simulink model "CCMLin2Mgr"  (R2022b)
settings: Solver=FixedStepDiscrete  StartTime=0.0  StopTime=10.0  FixedStep=0.02  RelTol=1e-3
undefined symbols (not defined in the file or in its folder): ComChlType, NetworkChlType, P_LinIfSchHandleType, P_Std_ReturnType
(part 1 of 3)
settings Model: Creator=blue.ge  LastModifiedBy=blue.ge
settings DataIOCC: SaveFormat=StructureWithTime
settings OptimizationCC: DefaultParameterBehavior=Inlined  EnhancedBackFolding=on  PassReuseOutputArgsAs=Individual arguments  ZeroExternalMemoryAtStartup=off  ZeroInternalMemoryAtStartup=off  OptimizeBlockOrder=speed  DifferentSizesBufferReuse=on  OptimizationCustomize=off  EfficientTunableParamExpr=on
settings DebuggingCC: ParameterTunabilityLossMsg=error
settings ModelReferenceCC: ModelReferenceNumInstancesAllowed=Single
settings SFSimCC: MATLABDynamicMemAlloc=off
settings RTWCC: Description=AUTOSAR  SystemTargetFile=autosar.tlc  GenCodeOnly=on  TemplateMakefile=ert_default_tmf
settings CodeAppCC: ForceParamTrailComments=on  IgnoreCustomStorageClasses=off  OperatorAnnotations=on  SimulinkDataObjDesc=on  SFDataObjDesc=on  InsertBlockDesc=on
settings STFCustomTargetCC: TargetLangStandard=C99 (ISO)  MultiwordLength=256  ParMdlRefBuildCompliant=on  CompOptLevelCompliant=on  IncludeMdlTerminateFcn=off  CombineOutputUpdateFcns=on  SuppressErrorStatus=on  ERTCustomFileBanners=on  SupportNonFinite=off  SupportComplex=off  SupportContinuousTime=off  SupportNonInlinedSFcns=off  RemoveResetFunc=on  MATLABClassNameForMDSCustomization=Simulink.SoftwareTarget.ERTCustomization  AutosarCompliant=on  UseToolchainInfoCompliant=on  LookupTableObjectStructAxisOrder=2,1,3,4,...  LUTObjectStructOrderEvenSpacing=Size,Table,Breakpoints  SystemTargetFile=autosar.tlc
settings ERTTargetCC: TargetLangStandard=C99 (ISO)  ConcurrentExecutionCompliant=off  IncludeMdlTerminateFcn=off  SuppressErrorStatus=on  SupportNonFinite=off  SupportComplex=off  ModelStepFunctionPrototypeControlCompliant=off  CPPClassGenCompliant=off  AutosarCompliant=on  LookupTableObjectStructAxisOrder=2,1,3,4,...  LUTObjectStructOrderEvenSpacing=Size,Table,Breakpoints  InlinedParameterPlacement=NonHierarchical  ERTCustomFileTemplate=example_file_process.tlc

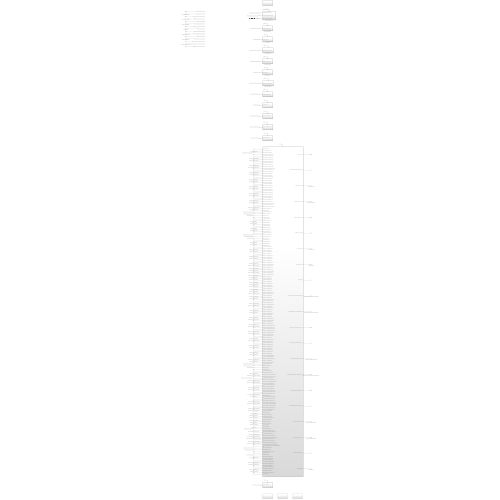
[thumbnail: Simulink's stored preview of the top-level diagram]
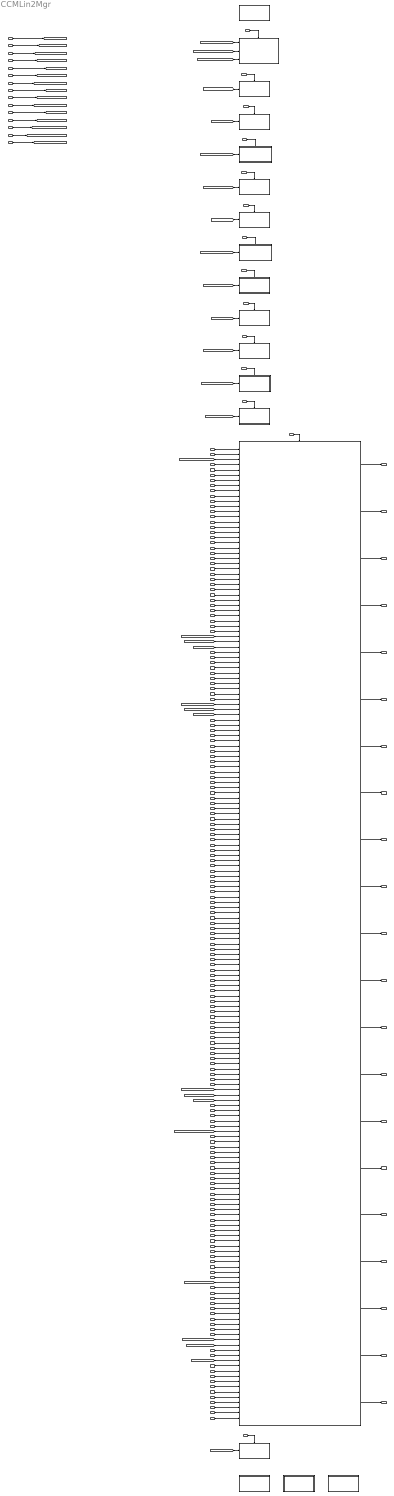
== system CCMLin2Mgr ==
  Lin2Mgr_AFUPartNo10Cmpl : Inport  OutputFunctionCall=on
  Lin2Mgr_AFUPartNoCmpl : Inport  Port=2  OutputFunctionCall=on
  Lin2Mgr_AFUSerNo : Inport  Port=3  OutputFunctionCall=on
  Lin2Mgr_AIUPartNo10Cmpl : Inport  Port=4  OutputFunctionCall=on
  Lin2Mgr_AIUPartNoCmpl : Inport  Port=5  OutputFunctionCall=on
  Lin2Mgr_AIUSerNo : Inport  Port=6  OutputFunctionCall=on
  Lin2Mgr_CDSPartNo10Cmpl : Inport  Port=7  OutputFunctionCall=on
  Lin2Mgr_CDSPartNoCmpl : Inport  Port=8  OutputFunctionCall=on
  Lin2Mgr_CDSSerNo : Inport  Port=9  OutputFunctionCall=on
  Lin2Mgr_KeepComActv : Inport  Port=10  SampleTime=0.02  OutputFunctionCall=on
  Lin2Mgr_PMSIPartNo10Hw : Inport  Port=11  OutputFunctionCall=on
  Lin2Mgr_PMSIPartNoHw : Inport  Port=12  OutputFunctionCall=on
  Lin2Mgr_Runnable : Inport  Port=13  SampleTime=0.1  OutputFunctionCall=on
  Lin2Mgr_SerNoPMSI : Inport  Port=14  OutputFunctionCall=on
  AFUPartNo10Cmpl_AFUPartNo10Cmpl : Inport  Port=15  OutDataTypeStr=Bus: PartNrNod10  PortDimensions=1  BusOutputAsStruct=on
  HvacCfgForClimaCtrl_HvacCfgForAQSTypes : Inport  Port=16  OutDataTypeStr=UInt8  PortDimensions=1
  SysServCarCfgSts_SysServCCPAQSSts : Inport  Port=17  OutDataTypeStr=UInt8  PortDimensions=1
  AFUPartNoCmpl_AFUPartNoCmpl : Inport  Port=18  OutDataTypeStr=Bus: PartNrNodLIN  PortDimensions=1  BusOutputAsStruct=on
  AFUSerNo_AFUSerNo : Inport  Port=19  OutDataTypeStr=Bus: NrSerlNodLIN  PortDimensions=1  BusOutputAsStruct=on
  AIUPartNo10Cmpl_AIUPartNo10Cmpl : Inport  Port=20  OutDataTypeStr=Bus: PartNrNod10  PortDimensions=1  BusOutputAsStruct=on
  AIUPartNoCmpl_AIUPartNoCmpl : Inport  Port=21  OutDataTypeStr=Bus: PartNrNodLIN  PortDimensions=1  BusOutputAsStruct=on
  AIUSerNo_AIUSerNo : Inport  Port=22  OutDataTypeStr=Bus: NrSerlNodLIN  PortDimensions=1  BusOutputAsStruct=on
  CDSPartNo10Cmpl_CDSPartNo10Cmpl : Inport  Port=23  OutDataTypeStr=Bus: PartNrNod10  PortDimensions=1  BusOutputAsStruct=on
  CDSPartNoCmpl_CDSPartNoCmpl : Inport  Port=24  OutDataTypeStr=Bus: PartNrNodLIN  PortDimensions=1  BusOutputAsStruct=on
  CDSSerNo_CDSSerNo : Inport  Port=25  OutDataTypeStr=Bus: NrSerlNodLIN  PortDimensions=1  BusOutputAsStruct=on
  KeepComActvL2_KeepComActvL2 : Inport  Port=26  OutDataTypeStr=Enum: OnOff1  PortDimensions=1
  PMSIPartNo10Hw_PMSIPartNo10Hw : Inport  Port=27  OutDataTypeStr=Bus: PartNrNod10  PortDimensions=1  BusOutputAsStruct=on
  PMSIPartNoHw_PMSIPartNoHw : Inport  Port=28  OutDataTypeStr=Bus: PartNrNodLIN  PortDimensions=1  BusOutputAsStruct=on
  RlyCmdCmft_RlyCmdCmft : Inport  Port=29  OutDataTypeStr=Enum: OnOff1  PortDimensions=1
  SysServCarCfgSts_SysServCarID : Inport  Port=30  OutDataTypeStr=Enum: SysServCarID  PortDimensions=1
  SysServCarCfgSts_SysServCCPHEXSensorSts : Inport  Port=31  OutDataTypeStr=UInt8  PortDimensions=1
  SysServCarCfgSts_SysServCCPLin2AFUSts : Inport  Port=32  OutDataTypeStr=Boolean  PortDimensions=1
  SysServCarCfgSts_SysServCCPLin2AIUSts : Inport  Port=33  OutDataTypeStr=UInt8  PortDimensions=1
  SysServCarCfgSts_SysServCCPLin2CDSSts : Inport  Port=34  OutDataTypeStr=Boolean  PortDimensions=1
  SysServCarCfgSts_SysServCCPLin2PMSISts : Inport  Port=35  OutDataTypeStr=Boolean  PortDimensions=1
  SysServCarCfgSts_SysServCCPLin3VGASts : Inport  Port=36  OutDataTypeStr=Boolean  PortDimensions=1
  SysServCarCfgSts_SysServDUAirVentSnrSts : Inport  Port=37  OutDataTypeStr=Boolean  PortDimensions=1
  SysServCarCfgSts_SysServDUBlowerPwmSignSts : Inport  Port=38  OutDataTypeStr=Boolean  PortDimensions=1
  SysServCarCfgSts_SysServDUBlowerPwmSignTypeSts : Inport  Port=39  OutDataTypeStr=UInt8  PortDimensions=1
  SysServCarCfgSts_SysServDUBlowerPwmSts : Inport  Port=40  OutDataTypeStr=Boolean  PortDimensions=1
  SysServCarCfgSts_SysServDUDCDefrostSts : Inport  Port=41  OutDataTypeStr=UInt8  PortDimensions=1
  SysServCarCfgSts_SysServDUDCModeSts : Inport  Port=42  OutDataTypeStr=UInt8  PortDimensions=1
  SysServCarCfgSts_SysServDUDCRecSts : Inport  Port=43  OutDataTypeStr=UInt8  PortDimensions=1
  SysServCarCfgSts_SysServDUDCSModeSts : Inport  Port=44  OutDataTypeStr=UInt8  PortDimensions=1
  SysServCarCfgSts_SysServDUDCSTLSts : Inport  Port=45  OutDataTypeStr=UInt8  PortDimensions=1
  SysServCarCfgSts_SysServDUDCTLSts : Inport  Port=46  OutDataTypeStr=UInt8  PortDimensions=1
  SysServCarCfgSts_SysServDUDCTRSts : Inport  Port=47  OutDataTypeStr=UInt8  PortDimensions=1
  SysServCarCfgSts_SysServDUFLFootSts : Inport  Port=48  OutDataTypeStr=UInt8  PortDimensions=1
  SysServCarCfgSts_SysServDUFLVentSts : Inport  Port=49  OutDataTypeStr=UInt8  PortDimensions=1
  SysServCarCfgSts_SysServDUFRFootSts : Inport  Port=50  OutDataTypeStr=UInt8  PortDimensions=1
  SysServCarCfgSts_SysServDUFRVentSts : Inport  Port=51  OutDataTypeStr=UInt8  PortDimensions=1
  SysServCarCfgSts_SysServDUIncarMotorSts : Inport  Port=52  OutDataTypeStr=Boolean  PortDimensions=1
  SysServCarCfgSts_SysServDULin1HBMFSts : Inport  Port=53  OutDataTypeStr=Boolean  PortDimensions=1
  SysServCarCfgSts_SysServDULin4HFAFSts : Inport  Port=54  OutDataTypeStr=UInt32  PortDimensions=1
  SysServCarCfgSts_SysServDUSFLFootSts : Inport  Port=55  OutDataTypeStr=UInt8  PortDimensions=1
  SysServCarCfgSts_SysServDUSFLVentSts : Inport  Port=56  OutDataTypeStr=UInt8  PortDimensions=1
  SysServCarCfgSts_SysServDUSFRFootSts : Inport  Port=57  OutDataTypeStr=UInt8  PortDimensions=1
  SysServCarCfgSts_SysServDUSFRVentSts : Inport  Port=58  OutDataTypeStr=UInt8  PortDimensions=1
  SysServCarCfgSts_SysServDUSnsrForFCmptmtTSts : Inport  Port=59  OutDataTypeStr=UInt8  PortDimensions=1
  SysServCarCfgSts_SysServDUSnsrForRair : Inport  Port=60  OutDataTypeStr=UInt8  PortDimensions=1
  SysServCarCfgSts_SysServDUSnsrForRCmptmtTSts : Inport  Port=61  OutDataTypeStr=UInt8  PortDimensions=1
  AACAirClngErrSts_AACAirClngErrSts : Inport  Port=62  OutDataTypeStr=Enum: AACAirClngErrSts  PortDimensions=1
  AFUGenErr_AFUGenErr : Inport  Port=63  OutDataTypeStr=Enum: OnOff1  PortDimensions=1
  AirClngErrSts_AirClngErrSts : Inport  Port=64  OutDataTypeStr=Enum: AirClngErrSts  PortDimensions=1
  AirFragFanErr_AirFragFanErr : Inport  Port=65  OutDataTypeStr=Enum: Err1  PortDimensions=1
  AirFragMot1Err_AirFragMot1Err : Inport  Port=66  OutDataTypeStr=Enum: Err1  PortDimensions=1
  AirFragMot2Err_AirFragMot2Err : Inport  Port=67  OutDataTypeStr=Enum: Err1  PortDimensions=1
  AirFragMot3Err_AirFragMot3Err : Inport  Port=68  OutDataTypeStr=Enum: Err1  PortDimensions=1
  AirFragMot4Err_AirFragMot4Err : Inport  Port=69  OutDataTypeStr=Enum: Err1  PortDimensions=1
  AirFragMot5Err_AirFragMot5Err : Inport  Port=70  OutDataTypeStr=Enum: Err1  PortDimensions=1
  AirFragOvrVoltErr_AirFragOvrVoltErr : Inport  Port=71  OutDataTypeStr=Enum: Err1  PortDimensions=1
  AirFragTempErr_AirFragTempErr : Inport  Port=72  OutDataTypeStr=Enum: TempErr  PortDimensions=1
  AirFragUndVoltErr_AirFragUndVoltErr : Inport  Port=73  OutDataTypeStr=Enum: Err1  PortDimensions=1
  AQSAirQuality_AQSAirQuality : Inport  Port=74  OutDataTypeStr=Enum: AQSAirQuality  PortDimensions=1
  AQSCOxstatus_AQSCOxstatus : Inport  Port=75  OutDataTypeStr=Enum: AQSAirQuality  PortDimensions=1
  AQSNH3status_AQSNH3status : Inport  Port=76  OutDataTypeStr=Enum: AQSAirQuality  PortDimensions=1
  AQSNoxStatus_AQSNoxStatus : Inport  Port=77  OutDataTypeStr=Enum: AQSAirQuality  PortDimensions=1
  CarCfgForClimaCtrl_CarCfgForAirClng : Inport  Port=78  OutDataTypeStr=Enum: CarCfgForAirClng  PortDimensions=1
  CarCfgForClimaCtrl_CarCfgForAirClnrAdv : Inport  Port=79  OutDataTypeStr=UInt8  PortDimensions=1
  CarCfgForClimaCtrl_CarCfgForAirFrag : Inport  Port=80  OutDataTypeStr=Enum: CarCfgForAirFrag  PortDimensions=1
  CarCfgForClimaCtrl_CarCfgForAirMtrlSnsr : Inport  Port=81  OutDataTypeStr=Enum: CarCfgForAirMtrlSnsrGly  PortDimensions=1
  CarCfgForClimaCtrl_CarCfgForAirQly : Inport  Port=82  OutDataTypeStr=Enum: CarCfgForAirQlyGly  PortDimensions=1
  CarCfgForClimaCtrl_CarCfgForAmntOfSeat : Inport  Port=83  OutDataTypeStr=Enum: CarCfgForAmntOfSeatTyp3  PortDimensions=1
  CarCfgForClimaCtrl_CarCfgForAutDemst : Inport  Port=84  OutDataTypeStr=UInt8  PortDimensions=1
  CarCfgForClimaCtrl_CarCfgForAuxWtrPmp : Inport  Port=85  OutDataTypeStr=Enum: CarCfgForAuxWtrPmp  PortDimensions=1
  CarCfgForClimaCtrl_CarCfgForBRAND : Inport  Port=86  OutDataTypeStr=UInt8  PortDimensions=1
  CarCfgForClimaCtrl_CarCfgForBypVlvInHvac : Inport  Port=87  OutDataTypeStr=Enum: CarCfgForBypVlvInHvac2  PortDimensions=1
  CarCfgForClimaCtrl_CarCfgForCabinOxgenSupply : Inport  Port=88  OutDataTypeStr=UInt8  PortDimensions=1
  CarCfgForClimaCtrl_CarCfgForClsVlvInHvac : Inport  Port=89  OutDataTypeStr=Enum: CarCfgForClsVlvInHvac  PortDimensions=1
  CarCfgForClimaCtrl_CarCfgForCmprAcTyp : Inport  Port=90  OutDataTypeStr=UInt8  PortDimensions=1
  CarCfgForClimaCtrl_CarCfgForCmptmtClng : Inport  Port=91  OutDataTypeStr=Enum: CarCfgForCmptmtClng  PortDimensions=1
  CarCfgForClimaCtrl_CarCfgForElectricLiftingModule : Inport  Port=92  OutDataTypeStr=UInt8  PortDimensions=1
  CarCfgForClimaCtrl_CarCfgForElecVentAdjmt : Inport  Port=93  OutDataTypeStr=Enum: CarCfgForElecVentAdjmt  PortDimensions=1
  CarCfgForClimaCtrl_CarCfgForExtVentnClimaCtrl : Inport  Port=94  OutDataTypeStr=Enum: CarCfgForExtVentnClimaCtrl  PortDimensions=1
  CarCfgForClimaCtrl_CarCfgForFlcTyp : Inport  Port=95  OutDataTypeStr=Enum: CarCfgForFlcTyp  PortDimensions=1
  CarCfgForClimaCtrl_CarCfgForFoldCSD : Inport  Port=96  OutDataTypeStr=Enum: CarCfgForFoldCSD  PortDimensions=1
  CarCfgForClimaCtrl_CarCfgForFuTyp : Inport  Port=97  OutDataTypeStr=Enum: CarCfgForFuTyp  PortDimensions=1
  CarCfgForClimaCtrl_CarCfgForGlassTyp : Inport  Port=98  OutDataTypeStr=Enum: CarCfgForGlassTyp  PortDimensions=1
  CarCfgForClimaCtrl_CarCfgForHeadRadar : Inport  Port=99  OutDataTypeStr=Enum: HeadRadar  PortDimensions=1
  CarCfgForClimaCtrl_CarCfgForHeatPmp : Inport  Port=100  OutDataTypeStr=Enum: CarCfgForHeatPmpTyp  PortDimensions=1
  CarCfgForClimaCtrl_CarCfgForHeatrDurgDrvg : Inport  Port=101  OutDataTypeStr=Enum: CarCfgForHeatrDurgDrvg  PortDimensions=1
  CarCfgForClimaCtrl_CarCfgForHeatrTyp : Inport  Port=102  OutDataTypeStr=UInt8  PortDimensions=1
  CarCfgForClimaCtrl_CarCfgForHvacFilTyp : Inport  Port=103  OutDataTypeStr=Enum: CarCfgForHvacFilTypGly  PortDimensions=1
  CarCfgForClimaCtrl_CarCfgForHvacFlrSnsrForT : Inport  Port=104  OutDataTypeStr=Enum: CarCfgForHvacFlrSnsrForT  PortDimensions=1
  CarCfgForClimaCtrl_CarCfgForHvacHeatrSnsrT : Inport  Port=105  OutDataTypeStr=Enum: CarCfgForHvacHeatrSnsrT  PortDimensions=1
  CarCfgForClimaCtrl_CarCfgForHvacRe : Inport  Port=106  OutDataTypeStr=UInt8  PortDimensions=1
  CarCfgForClimaCtrl_CarCfgForHvacTyp : Inport  Port=107  OutDataTypeStr=UInt8  PortDimensions=1
  CarCfgForClimaCtrl_CarCfgForInteriorViewCamera : Inport  Port=108  OutDataTypeStr=Enum: CarCfgForInteriorViewCamera  PortDimensions=1
  CarCfgForClimaCtrl_CarCfgForLifeDetection : Inport  Port=109  OutDataTypeStr=Enum: LifeDetection  PortDimensions=1
  CarCfgForClimaCtrl_CarCfgForLoVoltElecCfg : Inport  Port=110  OutDataTypeStr=Enum: CarCfgForLoVoltElecCfg  PortDimensions=1
  CarCfgForClimaCtrl_CarCfgForNavSysandIntnmap : Inport  Port=111  OutDataTypeStr=Enum: CarCfgForNavSysandIntnmap  PortDimensions=1
  CarCfgForClimaCtrl_CarCfgForNrOfDoor : Inport  Port=112  OutDataTypeStr=Enum: CarCfgForNrOfDoor2  PortDimensions=1
  CarCfgForClimaCtrl_CarCfgForPipeInFuTank : Inport  Port=113  OutDataTypeStr=Enum: CarCfgForPipeInFuTank  PortDimensions=1
  CarCfgForClimaCtrl_CarCfgForPowerSeats : Inport  Port=114  OutDataTypeStr=Enum: CarCfgForPowerSeats  PortDimensions=1
  CarCfgForClimaCtrl_CarCfgForPreClng : Inport  Port=115  OutDataTypeStr=Enum: CarCfgForPreClng  PortDimensions=1
  CarCfgForClimaCtrl_CarCfgForPrkgCDSNotAvl : Inport  Port=116  OutDataTypeStr=Enum: CarCfgForPrkgCDSNotAvl  PortDimensions=1
  CarCfgForClimaCtrl_CarCfgForPrkgClima : Inport  Port=117  OutDataTypeStr=Enum: CarCfgForPrkgClima  PortDimensions=1
  CarCfgForClimaCtrl_CarCfgForPrpsnTyp : Inport  Port=118  OutDataTypeStr=Enum: CarCfgForPrpsnTypGly  PortDimensions=1
  CarCfgForClimaCtrl_CarCfgForPTCInFrontHVAC : Inport  Port=119  OutDataTypeStr=UInt8  PortDimensions=1
  CarCfgForClimaCtrl_CarCfgForRemEngStrtPreCdn : Inport  Port=120  OutDataTypeStr=Enum: CarCfgForRemEngStrtPreCdn  PortDimensions=1
  CarCfgForClimaCtrl_CarCfgForRoofHatch : Inport  Port=121  OutDataTypeStr=Enum: CarCfgForRoofHatch  PortDimensions=1
  CarCfgForClimaCtrl_CarCfgForSeatHeatgCal : Inport  Port=122  OutDataTypeStr=Enum: CarCfgForSeatHeatgCal1  PortDimensions=1
  CarCfgForClimaCtrl_CarCfgForSeatHeatgForRowFirst : Inport  Port=123  OutDataTypeStr=Enum: CarCfgForSeatHeatgForRowFirst  PortDimensions=1
  CarCfgForClimaCtrl_CarCfgForSeatHeatgForRowSec : Inport  Port=124  OutDataTypeStr=Enum: CarCfgForSeatHeatgForRowSec  PortDimensions=1
  CarCfgForClimaCtrl_CarCfgForSeatVentnForRowFirst : Inport  Port=125  OutDataTypeStr=Enum: CarCfgForSeatVentnForRowFirst1  PortDimensions=1
  CarCfgForClimaCtrl_CarCfgForSeatVentnForRowSec : Inport  Port=126  OutDataTypeStr=Enum: CarCfgForSeatVentnForRowSecGly  PortDimensions=1
  CarCfgForClimaCtrl_CarCfgForSecRowVentnCenter : Inport  Port=127  OutDataTypeStr=Enum: CarCfgForSecRowVentnCenter  PortDimensions=1
  CarCfgForClimaCtrl_CarCfgForSecRowVentnSide : Inport  Port=128  OutDataTypeStr=Enum: CarCfgForSecRowVentnSide  PortDimensions=1
  CarCfgForClimaCtrl_CarCfgForSmallFlowCyc : Inport  Port=129  OutDataTypeStr=Enum: CarCfgForSmallFlowCyc  PortDimensions=1
  CarCfgForClimaCtrl_CarCfgForSmkSnsr : Inport  Port=130  OutDataTypeStr=Enum: CarCfgForSmkSnsr  PortDimensions=1
  CarCfgForClimaCtrl_CarCfgForSnsrForCmptmtT : Inport  Port=131  OutDataTypeStr=Enum: CarCfgForSnsrForCmptmtT  PortDimensions=1
  CarCfgForClimaCtrl_CarCfgForSnsrHumPrsnt : Inport  Port=132  OutDataTypeStr=Enum: CarCfgForSnsrHumPrsnt  PortDimensions=1
  CarCfgForClimaCtrl_CarCfgForStartDevice : Inport  Port=133  OutDataTypeStr=Enum: StartDevice  PortDimensions=1
  CarCfgForClimaCtrl_CarCfgForSteerWhlHeatg : Inport  Port=134  OutDataTypeStr=UInt8  PortDimensions=1
  CarCfgForClimaCtrl_CarCfgForSteerWhlPosn : Inport  Port=135  OutDataTypeStr=Enum: CarCfgForSteerWhlPosn  PortDimensions=1
  CarCfgForClimaCtrl_CarCfgForSunSnsr : Inport  Port=136  OutDataTypeStr=Enum: CarCfgForSunSnsr  PortDimensions=1
  CarCfgForClimaCtrl_CarCfgForTelmNodTyp : Inport  Port=137  OutDataTypeStr=Enum: CarCfgForTelmNodTyp  PortDimensions=1
  CarCfgForClimaCtrl_CarCfgForThermMngt : Inport  Port=138  OutDataTypeStr=Enum: CarCfgForThermMngt  PortDimensions=1
  CarCfgForClimaCtrl_CarCfgForVehTyp : Inport  Port=139  OutDataTypeStr=Enum: CarCfgForVehTyp3  PortDimensions=1
  CarCfgForClimaCtrl_CarCfgForVentLiftMotorExiSts : Inport  Port=140  OutDataTypeStr=UInt32  PortDimensions=1
  CarCfgForClimaCtrl_CarCfgForVgaMotorExistSts : Inport  Port=141  OutDataTypeStr=CarCfgForVgaMotorExistSts  PortDimensions=1
  CarCfgForClimaCtrl_CarCfgForWadingAssistSystem : Inport  Port=142  OutDataTypeStr=UInt8  PortDimensions=1
  CarCfgForClimaCtrl_CarCfgForWindHeatd : Inport  Port=143  OutDataTypeStr=Enum: CarCfgForWindHeatd  PortDimensions=1
  CarCfgForClimaCtrl_CarCfgWindFollowHumanMov : Inport  Port=144  OutDataTypeStr=UInt8  PortDimensions=1
  ClimRlyCmd_ClimRlyCmd : Inport  Port=145  OutDataTypeStr=Enum: OnOff1  PortDimensions=1
  CmpmtInAirPmAQI_CmpmtInAirPmAQI : Inport  Port=146  OutDataTypeStr=Enum: CmpmtAirPmLvl  PortDimensions=1
  CmpmtInAirPmDnsty_CmpmtInAirPmDnsty : Inport  Port=147  OutDataTypeStr=UInt10  PortDimensions=1
  CmptmtOxygenConcQFNgcc_CmptmtOxygenConcQFNgcc : Inport  Port=148  OutDataTypeStr=Enum: Qf1  PortDimensions=1
  CmptmtOxygenConcVluNgcc_CmptmtOxygenConcVluNgcc : Inport  Port=149  OutMin=0  OutMax=100  OutDataTypeStr=Perc9  PortDimensions=1
  HvacCfgForClimaCtrl_HvacCfgForHvacFrontBlowerType : Inport  Port=150  OutDataTypeStr=HvacCfgForHvacFrontBlowerType  PortDimensions=1
  HvacCfgForClimaCtrl_HvacCfgForHvacLowSideRETXVESts : Inport  Port=151  OutDataTypeStr=UInt8  PortDimensions=1
  HvacCfgForClimaCtrl_HvacCfgForHvacMiscExistSts : Inport  Port=152  OutDataTypeStr=HvacCfgForHvacMiscExistSts  PortDimensions=1
  HvacCfgForClimaCtrl_HvacCfgForHvacMotorExistSts : Inport  Port=153  OutDataTypeStr=HvacCfgForHvacMotorExistSts  PortDimensions=1
  HvacCfgForClimaCtrl_HvacCfgForHvacReBlowerType : Inport  Port=154  OutDataTypeStr=UInt8  PortDimensions=1
  HvacCfgForClimaCtrl_HvacCfgForHvacReVentPstn : Inport  Port=155  OutDataTypeStr=UInt8  PortDimensions=1
  HvacCfgForClimaCtrl_HvacCfgForHvacSensorExistSts : Inport  Port=156  OutDataTypeStr=HvacCfgForHvacSensorExistSts  PortDimensions=1
  HvacCfgForClimaCtrl_HvacCfgForHvacSnsrRCmptmtESts : Inport  Port=157  OutDataTypeStr=UInt8  PortDimensions=1
  HvacCfgForClimaCtrl_HvacCfgForHvacSnsrReHexESts : Inport  Port=158  OutDataTypeStr=UInt8  PortDimensions=1
  HvacFanReqRe_HvacFanReqRe : Inport  Port=159  OutDataTypeStr=HvacFanSpd  PortDimensions=1
  HvacFanSts2HvacFanBattU2_HvacFanSts2HvacFanBattU2 : Inport  Port=160  OutMin=0  OutMax=25.400000000000002  OutDataTypeStr=U8  PortDimensions=1
  HvacFanSts2HvacFanExt2_HvacFanSts2HvacFanExt2 : Inport  Port=161  OutDataTypeStr=UInt8  PortDimensions=1
  HvacFanSts2HvacFanHw2_HvacFanSts2HvacFanHw2 : Inport  Port=162  OutMin=0  OutMax=15  OutDataTypeStr=UInt4  PortDimensions=1
  HvacFanSts2HvacFanMotU2_HvacFanSts2HvacFanMotU2 : Inport  Port=163  OutMin=0  OutMax=25.400000000000002  OutDataTypeStr=U8  PortDimensions=1
  HvacFanSts2HvacFanReSts1_HvacFanSts2HvacFanReSts1 : Inport  Port=164  OutDataTypeStr=Enum: BlowerReErrSts  PortDimensions=1
  HvacFanSts2HvacFanReSts2_HvacFanSts2HvacFanReSts2 : Inport  Port=165  OutDataTypeStr=Enum: BlowerReSts  PortDimensions=1
  HvacFanSts2HvacFanSw2_HvacFanSts2HvacFanSw2 : Inport  Port=166  OutMin=0  OutMax=15  OutDataTypeStr=UInt4  PortDimensions=1
  HvacFanSts2HvacFanT2_HvacFanSts2HvacFanT2 : Inport  Port=167  OutDataTypeStr=T6  PortDimensions=1
  HvacFanVoltReqRe_HvacFanVoltReqRe : Inport  Port=168  OutMin=0  OutMax=100  OutDataTypeStr=Perc9  PortDimensions=1
  InPm25FanErr_InPm25FanErr : Inport  Port=169  OutDataTypeStr=Enum: FanErr  PortDimensions=1
  InPm25SensrIntErr_InPm25SensrIntErr : Inport  Port=170  OutDataTypeStr=Enum: VentnActr01UrgcExist  PortDimensions=1
  InPm25SnrTempErr_InPm25SnrTempErr : Inport  Port=171  OutDataTypeStr=Enum: TempErr  PortDimensions=1
  InPm25SnrVoltgRangErr_InPm25SnrVoltgRangErr : Inport  Port=172  OutDataTypeStr=Enum: PmSnrVoltgRangErr  PortDimensions=1
  InPm25SnsrElecErr_InPm25SnsrElecErr : Inport  Port=173  OutDataTypeStr=Enum: PmSnsrErrSts  PortDimensions=1
  InPm25SnsrSts_InPm25SnsrSts : Inport  Port=174  OutDataTypeStr=Enum: PmSnsrSts  PortDimensions=1
  IntCo2CircElecErr_IntCo2CircElecErr : Inport  Port=175  OutDataTypeStr=Enum: PmSnsrErrSts  PortDimensions=1
  IntCo2HvErr_IntCo2HvErr : Inport  Port=176  OutDataTypeStr=Enum: Err1  PortDimensions=1
  IntCo2LvErr_IntCo2LvErr : Inport  Port=177  OutDataTypeStr=Enum: Err1  PortDimensions=1
  OxygenConcSnsrElecErr_OxygenConcSnsrElecErr : Inport  Port=178  OutDataTypeStr=Enum: OxygenConcSnsrElecErr  PortDimensions=1
  OxygenConcSnsrElecErrNgcc_OxygenConcSnsrElecErrNgcc : Inport  Port=179  OutDataTypeStr=Enum: OxygenConcSnsrElecErr  PortDimensions=1
  OxygenConcSnsrIntErr_OxygenConcSnsrIntErr : Inport  Port=180  OutDataTypeStr=Enum: Err1  PortDimensions=1
  OxygenConcSnsrIntErrNgcc_OxygenConcSnsrIntErrNgcc : Inport  Port=181  OutDataTypeStr=Enum: Err1  PortDimensions=1
  OxygenConcSnsrOvrVoltRangErr_OxygenConcSnsrOvrVoltRangErr : Inport  Port=182  OutDataTypeStr=Enum: Err1  PortDimensions=1
  OxygenConcSnsrStsNgcc_OxygenConcSnsrStsNgcc : Inport  Port=183  OutDataTypeStr=Enum: PmSnsrSts  PortDimensions=1
  OxygenConcSnsrTempErr_OxygenConcSnsrTempErr : Inport  Port=184  OutDataTypeStr=Enum: TempErr  PortDimensions=1
  OxygenConcSnsrTempErrNgcc_OxygenConcSnsrTempErrNgcc : Inport  Port=185  OutDataTypeStr=Enum: TempErr  PortDimensions=1
  OxygenConcSnsrUndVoltRangErr_OxygenConcSnsrUndVoltRangErr : Inport  Port=186  OutDataTypeStr=Enum: Err1  PortDimensions=1
  OxygenConcSnsrUndVoltRangErrNgcc_OxygenConcSnsrUndVoltRangErrNgcc : Inport  Port=187  OutDataTypeStr=Enum: Err1  PortDimensions=1
  PwrSplyToSnsrForAirQly_PwrSplyToSnsrForAirQly : Inport  Port=188  OutDataTypeStr=Enum: OnOff1  PortDimensions=1
  RHFAScheTableReq_Val : Inport  Port=189  OutDataTypeStr=UInt8  PortDimensions=1
  SerNoPMSI_SerNoPMSI : Inport  Port=190  OutDataTypeStr=Bus: NrSerlNodLIN  PortDimensions=1  BusOutputAsStruct=on
  SysServCarCfgSts_SysServCCPBGMNum : Inport  Port=191  OutDataTypeStr=UInt8  PortDimensions=1
  SysServCarCfgSts_SysServCCPLin2AQSSts : Inport  Port=192  OutDataTypeStr=Boolean  PortDimensions=1
  SysServCarCfgSts_SysServDUDCFLMdeSts : Inport  Port=193  OutDataTypeStr=UInt8  PortDimensions=1
  SysServCarCfgSts_SysServDUReBlowerPwmSignSts : Inport  Port=194  OutDataTypeStr=UInt8  PortDimensions=1
  SysServCarCfgSts_SysServDUReBlowerPwmSts : Inport  Port=195  OutDataTypeStr=UInt8  PortDimensions=1
  SysServCarCfgSts_SysServFrntHEXSnsrPosn : Inport  Port=196  OutDataTypeStr=UInt8  PortDimensions=1
  SysServCarCfgSts_SysServLowSideRETXVSts : Inport  Port=197  OutDataTypeStr=UInt8  PortDimensions=1
  SysServCarCfgSts_SysServReHEXSnsrPosn : Inport  Port=198  OutDataTypeStr=UInt8  PortDimensions=1
  SysServCarCfgSts_SysServRHFASts : Inport  Port=199  OutDataTypeStr=UInt8  PortDimensions=1
  SysServCarCfgSts_SysServSnsrForFCmptmtTType : Inport  Port=200  OutDataTypeStr=UInt8  PortDimensions=1
  BswLifeCycle_LifeCycle : Inport  Port=201  OutDataTypeStr=Enum: CCMLifeCycleModeGroup  PortDimensions=1
  Lin2MgrDtcServ : Subsystem
  Lin2MgrParaServ : Subsystem
  Lin2Mgr_AFUPartNo10Cmpl_sys : Subsystem
  Lin2Mgr_AFUPartNoCmpl_sys : Subsystem
  Lin2Mgr_AFUSerNo_sys : Subsystem
  Lin2Mgr_AIUPartNo10Cmpl_sys : Subsystem
  Lin2Mgr_AIUPartNoCmpl_sys : Subsystem
  Lin2Mgr_AIUSerNo_sys : Subsystem
  Lin2Mgr_CDSPartNo10Cmpl_sys : Subsystem
  Lin2Mgr_CDSPartNoCmpl_sys : Subsystem
  Lin2Mgr_CDSSerNo_sys : Subsystem
  Lin2Mgr_Init : Subsystem
  Lin2Mgr_KeepComActv_sys : Subsystem
  Lin2Mgr_PMSIPartNo10Hw_sys : Subsystem
  Lin2Mgr_PMSIPartNoHw_sys : Subsystem
  Lin2Mgr_Runnable_sys : Subsystem
  Lin2Mgr_SerNoPMSI_sys : Subsystem
  Lin2ParaService : Subsystem
  AirQlyLin_AirQlyLin : Outport  OutDataTypeStr=Bus: AirQlyLinDutyWithQly  PortDimensions=1  BusOutputAsStruct=on
  HvacFanSet2HvacFanRxFrq2_HvacFanSet2HvacFanRxFrq2 : Outport  Port=2  OutMin=150  OutMax=4245  OutDataTypeStr=BlowerReFrq  PortDimensions=1
  Lin2ActvReady_Lin2ActvReady : Outport  Port=3  OutDataTypeStr=UInt8  PortDimensions=1
  Lin2MgrDtcServ2_AACGenElectrFail : Outport  Port=4  OutDataTypeStr=UInt8  PortDimensions=1
  Lin2MgrDtcServ2_IOSMGenElectrFail : Outport  Port=5  OutDataTypeStr=UInt8  PortDimensions=1
  Lin2MgrDtcServ2_IOSMInterFail : Outport  Port=6  OutDataTypeStr=UInt8  PortDimensions=1
  ReVbmrSts_ReVbmrSts : Outport  Port=7  OutDataTypeStr=Enum: HvacFanSts  PortDimensions=1
  AirFragErrSts_AirFragErrSts : Outport  Port=8  OutDataTypeStr=Boolean  PortDimensions=1
  AirPmQf_AirPmQf : Outport  Port=9  OutDataTypeStr=Enum: AirPmQf  PortDimensions=1
  HvacFanSet2HvacFanIMaxMax2_HvacFanSet2HvacFanIMaxMax2 : Outport  Port=10  OutDataTypeStr=Ampr2  PortDimensions=1
  HvacFanSet2HvacFanIMaxMin2_HvacFanSet2HvacFanIMaxMin2 : Outport  Port=11  OutDataTypeStr=Ampr3  PortDimensions=1
  HvacFanSet2HvacFanKneeU2_HvacFanSet2HvacFanKneeU2 : Outport  Port=12  OutDataTypeStr=U53  PortDimensions=1
  HvacFanSet2HvacFanRamp2_HvacFanSet2HvacFanRamp2 : Outport  Port=13  OutDataTypeStr=SpdURamp2  PortDimensions=1
  HvacFanSet2HvacFanSeln2_HvacFanSet2HvacFanSeln2 : Outport  Port=14  OutDataTypeStr=Enum: HvacFanSeln  PortDimensions=1
  HvacFanSet2HvacFanSftyRunThd2_HvacFanSet2HvacFanSftyRunThd2 : Outport  Port=15  OutDataTypeStr=HvacFanSftyRunThd  PortDimensions=1
  HvacFanSet2HvacFanSpd2_HvacFanSet2HvacFanSpd2 : Outport  Port=16  OutDataTypeStr=UInt8  PortDimensions=1
  HvacFanSet2HvacSftyRunSpd2_HvacFanSet2HvacSftyRunSpd2 : Outport  Port=17  OutDataTypeStr=UInt8  PortDimensions=1
  IntPm25LvlFrmClima_IntPm25LvlFrmClima : Outport  Port=18  OutDataTypeStr=Enum: CmpmtAirPmLvl  PortDimensions=1